simulink model "complex_iq_bw2048_out32_fixaxi_v12b"  (R2019a) (continued)
== system complex_iq_bw2048_out32_fixaxi_v12b == (continued)
      mask DialogControl t224_DualTileConfig Prompt: Dual Tile Configuration
      mask DialogControl t224_DT_adc0 Prompt: ADC 0
      mask DialogControl t224_DT_adc0_DataSettings Prompt: Data Settings
      mask DialogControl t224_DT_adc0_req_axis_clk_label Prompt: Required AXI4-Stream clock:
      mask DialogControl t224_DT_adc0_MixerSettings Prompt: MIxer Settings
      mask DialogControl t224_DT_adc0_AnalogSettings Prompt: Analog Settings
      mask DialogControl t224_DT_adc1 Prompt: ADC 1
      mask DialogControl t224_DT_adc1_DataSettings Prompt: Data Settings
      mask DialogControl t224_DT_adc1_req_axis_clk_label Prompt: Required AXI4-Stream clock:
      mask DialogControl t224_DT_adc1_MixerSettings Prompt: MIxer Settings
      mask DialogControl t224_DT_adc1_AnalogSettings Prompt: Analog Settings
      mask DialogControl Tile225_container Prompt: Tile 225
      mask DialogControl pll_clocking_config_225 Prompt: Clocking and PLL Configuration
      mask DialogControl t225_QuadTileConfig Prompt: Quad Tile Configuration
      mask DialogControl t225_ADCPair01 Prompt: ADC Pair 0,1
      mask DialogControl t225_QT_adc0 Prompt: ADC 0
      mask DialogControl t225_QT_adc0_DataSettings Prompt: Data Settings
      mask DialogControl t225_QT_adc0_req_axis_clk_label Prompt: Required AXI4-Stream clock:
      mask DialogControl t225_QT_adc0_MixerSettings Prompt: MIxer Settings
      mask DialogControl t225_QT_adc0_AnalogSettings Prompt: Analog Settings
      mask DialogControl t225_QT_adc1 Prompt: ADC 1
      mask DialogControl t225_QT_adc1_DataSettings Prompt: Data Settings
      mask DialogControl t225_QT_adc1_req_axis_clk_label Prompt: Required AXI4-Stream clock:
      mask DialogControl t225_QT_adc1_MixerSettings Prompt: MIxer Settings
      mask DialogControl t225_QT_adc1_AnalogSettings Prompt: Analog Settings
      mask DialogControl t225_ADCPair23 Prompt: ADC Pair 2,3
      mask DialogControl t225_QT_adc2 Prompt: ADC 2
      mask DialogControl t225_QT_adc2_DataSettings Prompt: Data Settings
      mask DialogControl t225_QT_adc2_req_axis_clk_label Prompt: Required AXI4-Stream clock:
      mask DialogControl t225_QT_adc2_MixerSettings Prompt: MIxer Settings
      mask DialogControl t225_QT_adc2_AnalogSettings Prompt: Analog Settings
      mask DialogControl t225_QT_adc3 Prompt: ADC 3
      mask DialogControl t225_QT_adc3_DataSettings Prompt: Data Settings
      mask DialogControl t225_QT_adc3_req_axis_clk_label Prompt: Required AXI4-Stream clock:
      mask DialogControl t225_QT_adc3_MixerSettings Prompt: MIxer Settings
      mask DialogControl t225_QT_adc3_AnalogSettings Prompt: Analog Settings
      mask DialogControl t225_DualTileConfig Prompt: Dual Tile Configuration
      mask DialogControl t225_DT_adc0 Prompt: ADC 0
      mask DialogControl t225_DT_adc0_DataSettings Prompt: Data Settings
      mask DialogControl t225_DT_adc0_req_axis_clk_label Prompt: Required AXI4-Stream clock:
      mask DialogControl t225_DT_adc0_MixerSettings Prompt: MIxer Settings
      mask DialogControl t225_DT_adc0_AnalogSettings Prompt: Analog Settings
      mask DialogControl t225_DT_adc1 Prompt: ADC 1
      mask DialogControl t225_DT_adc1_DataSettings Prompt: Data Settings
      mask DialogControl t225_DT_adc1_req_axis_clk_label Prompt: Required AXI4-Stream clock:
      mask DialogControl t225_DT_adc1_MixerSettings Prompt: MIxer Settings
      mask DialogControl t225_DT_adc1_AnalogSettings Prompt: Analog Settings
      mask DialogControl Tile226_container Prompt: Tile 226
      mask DialogControl pll_clocking_config_226 Prompt: Clocking and PLL Configuration
      mask DialogControl t226_QuadTileConfig Prompt: Quad Tile Configuration
      mask DialogControl t226_ADCPair01 Prompt: ADC Pair 0,1
      mask DialogControl t226_QT_adc0 Prompt: ADC 0
      mask DialogControl t226_QT_adc0_DataSettings Prompt: Data Settings
      mask DialogControl t226_QT_adc0_req_axis_clk_label Prompt: Required AXI4-Stream clock:
      mask DialogControl t226_QT_adc0_MixerSettings Prompt: MIxer Settings
      mask DialogControl t226_QT_adc0_AnalogSettings Prompt: Analog Settings
      mask DialogControl t226_QT_adc1 Prompt: ADC 1
      mask DialogControl t226_QT_adc1_DataSettings Prompt: Data Settings
      mask DialogControl t226_QT_adc1_req_axis_clk_label Prompt: Required AXI4-Stream clock:
      mask DialogControl t226_QT_adc1_MixerSettings Prompt: MIxer Settings
      mask DialogControl t226_QT_adc1_AnalogSettings Prompt: Analog Settings
      mask DialogControl t226_ADCPair23 Prompt: ADC Pair 2,3
      mask DialogControl t226_QT_adc2 Prompt: ADC 2
      mask DialogControl t226_QT_adc2_DataSettings Prompt: Data Settings
      mask DialogControl t226_QT_adc2_req_axis_clk_label Prompt: Required AXI4-Stream clock:
      mask DialogControl t226_QT_adc2_MixerSettings Prompt: MIxer Settings
      mask DialogControl t226_QT_adc2_AnalogSettings Prompt: Analog Settings
      mask DialogControl t226_QT_adc3 Prompt: ADC 3
      mask DialogControl t226_QT_adc3_DataSettings Prompt: Data Settings
      mask DialogControl t226_QT_adc3_req_axis_clk_label Prompt: Required AXI4-Stream clock:
      mask DialogControl t226_QT_adc3_MixerSettings Prompt: MIxer Settings
      mask DialogControl t226_QT_adc3_AnalogSettings Prompt: Analog Settings
      mask DialogControl t226_DualTileConfig Prompt: Dual Tile Configuration
      mask DialogControl t226_DT_adc0 Prompt: ADC 0
      mask DialogControl t226_DT_adc0_DataSettings Prompt: Data Settings
      mask DialogControl t226_DT_adc0_req_axis_clk_label Prompt: Required AXI4-Stream clock:
      mask DialogControl t226_DT_adc0_MixerSettings Prompt: MIxer Settings
      mask DialogControl t226_DT_adc0_AnalogSettings Prompt: Analog Settings
      mask DialogControl t226_DT_adc1 Prompt: ADC 1
      mask DialogControl t226_DT_adc1_DataSettings Prompt: Data Settings
      mask DialogControl t226_DT_adc1_req_axis_clk_label Prompt: Required AXI4-Stream clock:
      mask DialogControl t226_DT_adc1_MixerSettings Prompt: MIxer Settings
      mask DialogControl t226_DT_adc1_AnalogSettings Prompt: Analog Settings
      mask DialogControl Tile227_container Prompt: Tile 227
      mask DialogControl pll_clocking_config_227 Prompt: Clocking and PLL Configuration
      mask DialogControl t227_QuadTileConfig Prompt: Quad Tile Configuration
      mask DialogControl t227_ADCPair01 Prompt: ADC Pair 0,1
      mask DialogControl t227_QT_adc0 Prompt: ADC 0
      mask DialogControl t227_QT_adc0_DataSettings Prompt: Data Settings
      mask DialogControl t227_QT_adc0_req_axis_clk_label Prompt: Required AXI4-Stream clock:
      mask DialogControl t227_QT_adc0_MixerSettings Prompt: MIxer Settings
      mask DialogControl t227_QT_adc0_AnalogSettings Prompt: Analog Settings
      mask DialogControl t227_QT_adc1 Prompt: ADC 1
      mask DialogControl t227_QT_adc1_DataSettings Prompt: Data Settings
      mask DialogControl t227_QT_adc1_req_axis_clk_label Prompt: Required AXI4-Stream clock:
      mask DialogControl t227_QT_adc1_MixerSettings Prompt: MIxer Settings
      mask DialogControl t227_QT_adc1_AnalogSettings Prompt: Analog Settings
      mask DialogControl t227_ADCPair23 Prompt: ADC Pair 2,3
      mask DialogControl t227_QT_adc2 Prompt: ADC 2
      mask DialogControl t227_QT_adc2_DataSettings Prompt: Data Settings
      mask DialogControl t227_QT_adc2_req_axis_clk_label Prompt: Required AXI4-Stream clock:
      mask DialogControl t227_QT_adc2_MixerSettings Prompt: MIxer Settings
      mask DialogControl t227_QT_adc2_AnalogSettings Prompt: Analog Settings
      mask DialogControl t227_QT_adc3 Prompt: ADC 3
      mask DialogControl t227_QT_adc3_DataSettings Prompt: Data Settings
      mask DialogControl t227_QT_adc3_req_axis_clk_label Prompt: Required AXI4-Stream clock:
      mask DialogControl t227_QT_adc3_MixerSettings Prompt: MIxer Settings
      mask DialogControl t227_QT_adc3_AnalogSettings Prompt: Analog Settings
      mask DialogControl t227_DualTileConfig Prompt: Dual Tile Configuration
      mask DialogControl t227_DT_adc0 Prompt: ADC 0
      mask DialogControl t227_DT_adc0_DataSettings Prompt: Data Settings
      mask DialogControl t227_DT_adc0_req_axis_clk_label Prompt: Required AXI4-Stream clock:
      mask DialogControl t227_DT_adc0_MixerSettings Prompt: MIxer Settings
      mask DialogControl t227_DT_adc0_AnalogSettings Prompt: Analog Settings
      mask DialogControl t227_DT_adc1 Prompt: ADC 1
      mask DialogControl t227_DT_adc1_DataSettings Prompt: Data Settings
      mask DialogControl t227_DT_adc1_req_axis_clk_label Prompt: Required AXI4-Stream clock:
      mask DialogControl t227_DT_adc1_MixerSettings Prompt: MIxer Settings
      mask DialogControl t227_DT_adc1_AnalogSettings Prompt: Analog Settings
      mask DialogControl DACTileSelect Prompt: DAC Tiles
      mask DialogControl Tile228_container Prompt: Tile 228
      mask DialogControl pll_clocking_config_228 Prompt: Clocking and PLL Configuration
      mask DialogControl t228_QuadTileConfig Prompt: Quad Tile Configuration
      mask DialogControl t228_DACPair01 Prompt: DAC Pair 0,1
      mask DialogControl t228_QT_dac0 Prompt: DAC 0
      mask DialogControl t228_QT_dac0_DataSettings Prompt: Data Settings
      mask DialogControl t228_QT_dac0_req_axis_clk_label Prompt: Required AXI4-Stream clock:
      mask DialogControl t228_QT_dac0_MixerSettings Prompt: MIxer Settings
      mask DialogControl t228_QT_dac0_AnalogSettings Prompt: Analog Settings
      mask DialogControl t228_QT_dac1 Prompt: DAC 1
      mask DialogControl t228_QT_dac1_DataSettings Prompt: Data Settings
      mask DialogControl t228_QT_dac1_req_axis_clk_label Prompt: Required AXI4-Stream clock:
      mask DialogControl t228_QT_dac1_MixerSettings Prompt: MIxer Settings
      mask DialogControl t228_QT_dac1_AnalogSettings Prompt: Analog Settings
      mask DialogControl t228_DACPair23 Prompt: DAC Pair 2,3
      mask DialogControl t228_QT_dac2 Prompt: DAC 2
      mask DialogControl t228_QT_dac2_DataSettings Prompt: Data Settings
      mask DialogControl t228_QT_dac2_req_axis_clk_label Prompt: Required AXI4-Stream clock:
      mask DialogControl t228_QT_dac2_MixerSettings Prompt: MIxer Settings
      mask DialogControl t228_QT_dac2_AnalogSettings Prompt: Analog Settings
      mask DialogControl t228_QT_dac3 Prompt: DAC 3
      mask DialogControl t228_QT_dac3_DataSettings Prompt: Data Settings
      mask DialogControl t228_QT_dac3_req_axis_clk_label Prompt: Required AXI4-Stream clock:
      mask DialogControl t228_QT_dac3_MixerSettings Prompt: MIxer Settings
      mask DialogControl t228_QT_dac3_AnalogSettings Prompt: Analog Settings
      mask DialogControl t228_DualTileConfig Prompt: Dual Tile Configuration
      mask DialogControl t228_DT_dac0 Prompt: DAC 0
      mask DialogControl t228_DT_dac0_DataSettings Prompt: Data Settings
      mask DialogControl t228_DT_dac0_req_axis_clk_label Prompt: Required AXI4-Stream clock:
      mask DialogControl t228_DT_dac0_MixerSettings Prompt: MIxer Settings
      mask DialogControl t228_DT_dac0_AnalogSettings Prompt: Analog Settings
      mask DialogControl t228_DT_dac1 Prompt: DAC 1
      mask DialogControl t228_DT_dac1_DataSettings Prompt: Data Settings
      mask DialogControl t228_DT_dac1_req_axis_clk_label Prompt: Required AXI4-Stream clock:
      mask DialogControl t228_DT_dac1_MixerSettings Prompt: MIxer Settings
      mask DialogControl t228_DT_dac1_AnalogSettings Prompt: Analog Settings
      mask DialogControl Tile229_container Prompt: Tile 229
      mask DialogControl pll_clocking_config_229 Prompt: Clocking and PLL Configuration
      mask DialogControl t229_QuadTileConfig Prompt: Quad Tile Configuration
      mask DialogControl t229_DACPair01 Prompt: DAC Pair 0,1
      mask DialogControl t229_QT_dac0 Prompt: DAC 0
      mask DialogControl t229_QT_dac0_DataSettings Prompt: Data Settings
      mask DialogControl t229_QT_dac0_req_axis_clk_label Prompt: Required AXI4-Stream clock:
      mask DialogControl t229_QT_dac0_MixerSettings Prompt: MIxer Settings
      mask DialogControl t229_QT_dac0_AnalogSettings Prompt: Analog Settings
      mask DialogControl t229_QT_dac1 Prompt: DAC 1
      mask DialogControl t229_QT_dac1_DataSettings Prompt: Data Settings
      mask DialogControl t229_QT_dac1_req_axis_clk_label Prompt: Required AXI4-Stream clock:
      mask DialogControl t229_QT_dac1_MixerSettings Prompt: MIxer Settings
      mask DialogControl t229_QT_dac1_AnalogSettings Prompt: Analog Settings
      mask DialogControl t229_DACPair23 Prompt: DAC Pair 2,3
      mask DialogControl t229_QT_dac2 Prompt: DAC 2
      mask DialogControl t229_QT_dac2_DataSettings Prompt: Data Settings
      mask DialogControl t229_QT_dac2_req_axis_clk_label Prompt: Required AXI4-Stream clock:
      mask DialogControl t229_QT_dac2_MixerSettings Prompt: MIxer Settings
      mask DialogControl t229_QT_dac2_AnalogSettings Prompt: Analog Settings
      mask DialogControl t229_QT_dac3 Prompt: DAC 3
      mask DialogControl t229_QT_dac3_DataSettings Prompt: Data Settings
      mask DialogControl t229_QT_dac3_req_axis_clk_label Prompt: Required AXI4-Stream clock:
      mask DialogControl t229_QT_dac3_MixerSettings Prompt: MIxer Settings
      mask DialogControl t229_QT_dac3_AnalogSettings Prompt: Analog Settings
      mask DialogControl t229_DualTileConfig Prompt: Dual Tile Configuration
      mask DialogControl t229_DT_dac0 Prompt: DAC 0
      mask DialogControl t229_DT_dac0_DataSettings Prompt: Data Settings
      mask DialogControl t229_DT_dac0_req_axis_clk_label Prompt: Required AXI4-Stream clock:
      mask DialogControl t229_DT_dac0_MixerSettings Prompt: MIxer Settings
      mask DialogControl t229_DT_dac0_AnalogSettings Prompt: Analog Settings
      mask DialogControl t229_DT_dac1 Prompt: DAC 1
      mask DialogControl t229_DT_dac1_DataSettings Prompt: Data Settings
      mask DialogControl t229_DT_dac1_req_axis_clk_label Prompt: Required AXI4-Stream clock:
      mask DialogControl t229_DT_dac1_MixerSettings Prompt: MIxer Settings
      mask DialogControl t229_DT_dac1_AnalogSettings Prompt: Analog Settings
      mask DialogControl Tile230_container Prompt: Tile 230
      mask DialogControl pll_clocking_config_230 Prompt: Clocking and PLL Configuration
      mask DialogControl t230_QuadTileConfig Prompt: Quad Tile Configuration
      mask DialogControl t230_DACPair01 Prompt: DAC Pair 0,1
      mask DialogControl t230_QT_dac0 Prompt: DAC 0
      mask DialogControl t230_QT_dac0_DataSettings Prompt: Data Settings
      mask DialogControl t230_QT_dac0_req_axis_clk_label Prompt: Required AXI4-Stream clock:
      mask DialogControl t230_QT_dac0_MixerSettings Prompt: MIxer Settings
      mask DialogControl t230_QT_dac0_AnalogSettings Prompt: Analog Settings
      mask DialogControl t230_QT_dac1 Prompt: DAC 1
      mask DialogControl t230_QT_dac1_DataSettings Prompt: Data Settings
      mask DialogControl t230_QT_dac1_req_axis_clk_label Prompt: Required AXI4-Stream clock:
      mask DialogControl t230_QT_dac1_MixerSettings Prompt: MIxer Settings
      mask DialogControl t230_QT_dac1_AnalogSettings Prompt: Analog Settings
      mask DialogControl t230_DACPair23 Prompt: DAC Pair 2,3
      mask DialogControl t230_QT_dac2 Prompt: DAC 2
      mask DialogControl t230_QT_dac2_DataSettings Prompt: Data Settings
      mask DialogControl t230_QT_dac2_req_axis_clk_label Prompt: Required AXI4-Stream clock:
      mask DialogControl t230_QT_dac2_MixerSettings Prompt: MIxer Settings
      mask DialogControl t230_QT_dac2_AnalogSettings Prompt: Analog Settings
      mask DialogControl t230_QT_dac3 Prompt: DAC 3
      mask DialogControl t230_QT_dac3_DataSettings Prompt: Data Settings
      mask DialogControl t230_QT_dac3_req_axis_clk_label Prompt: Required AXI4-Stream clock:
      mask DialogControl t230_QT_dac3_MixerSettings Prompt: MIxer Settings
      mask DialogControl t230_QT_dac3_AnalogSettings Prompt: Analog Settings
      mask DialogControl t230_DualTileConfig Prompt: Dual Tile Configuration
      mask DialogControl t230_DT_dac0 Prompt: DAC 0
      mask DialogControl t230_DT_dac0_DataSettings Prompt: Data Settings
      mask DialogControl t230_DT_dac0_req_axis_clk_labe Prompt: Required AXI4-Stream clock:
      mask DialogControl t230_DT_dac0_MixerSettings Prompt: MIxer Settings
      mask DialogControl t230_DT_dac0_AnalogSettings Prompt: Analog Settings
      mask DialogControl t230_DT_dac1 Prompt: DAC 1
      mask DialogControl t230_DT_dac1_DataSettings Prompt: Data Settings
      mask DialogControl t230_DT_dac1_req_axis_clk_label Prompt: Required AXI4-Stream clock:
      mask DialogControl t230_DT_dac1_MixerSettings Prompt: MIxer Settings
      mask DialogControl t230_DT_dac1_AnalogSettings Prompt: Analog Settings
      mask DialogControl Tile231_container Prompt: Tile 231
      mask DialogControl pll_clocking_config_231 Prompt: Clocking and PLL Configuration
      mask DialogControl t231_QuadTileConfig Prompt: Quad Tile Configuration
      mask DialogControl t231_DACPair01 Prompt: DAC Pair 0,1
      mask DialogControl t231_QT_dac0 Prompt: DAC 0
      mask DialogControl t231_QT_dac0_DataSettings Prompt: Data Settings
      mask DialogControl t231_QT_dac0_req_axis_clk_label Prompt: Required AXI4-Stream clock:
      mask DialogControl t231_QT_dac0_MixerSettings Prompt: MIxer Settings
      mask DialogControl t231_QT_dac0_AnalogSettings Prompt: Analog Settings
      mask DialogControl t231_QT_dac1 Prompt: DAC 1
      mask DialogControl t231_QT_dac1_DataSettings Prompt: Data Settings
      mask DialogControl t231_QT_dac1_req_axis_clk_label Prompt: Required AXI4-Stream clock:
      mask DialogControl t231_QT_dac1_MixerSettings Prompt: MIxer Settings
      mask DialogControl t231_QT_dac1_AnalogSettings Prompt: Analog Settings
      mask DialogControl t231_DACPair23 Prompt: DAC Pair 2,3
      mask DialogControl t231_QT_dac2 Prompt: DAC 2
      mask DialogControl t231_QT_dac2_DataSettings Prompt: Data Settings
      mask DialogControl t231_QT_dac2_req_axis_clk_label Prompt: Required AXI4-Stream clock:
      mask DialogControl t231_QT_dac2_MixerSettings Prompt: MIxer Settings
      mask DialogControl t231_QT_dac2_AnalogSettings Prompt: Analog Settings
      mask DialogControl t231_QT_dac3 Prompt: DAC 3
      mask DialogControl t231_QT_dac3_DataSettings Prompt: Data Settings
      mask DialogControl t231_QT_dac3_req_axis_clk_label Prompt: Required AXI4-Stream clock:
      mask DialogControl t231_QT_dac3_MixerSettings Prompt: MIxer Settings
      mask DialogControl t231_QT_dac3_AnalogSettings Prompt: Analog Settings
      mask DialogControl t231_DualTileConfig Prompt: Dual Tile Configuration
      mask DialogControl t231_DT_dac0 Prompt: DAC 0
      mask DialogControl t231_DT_dac0_DataSettings Prompt: Data Settings
      mask DialogControl t231_DT_dac0_req_axis_clk_label Prompt: Required AXI4-Stream clock:
      mask DialogControl t231_DT_dac0_MixerSettings Prompt: MIxer Settings
      mask DialogControl t231_DT_dac0_AnalogSettings Prompt: Analog Settings
      mask DialogControl t231_DT_dac1 Prompt: DAC 1
      mask DialogControl t231_DT_dac1_DataSettings Prompt: Data Settings
      mask DialogControl t231_DT_dac1_req_axis_clk_label Prompt: Required AXI4-Stream clock:
      mask DialogControl t231_DT_dac1_MixerSettings Prompt: MIxer Settings
      mask DialogControl t231_DT_dac1_AnalogSettings Prompt: Analog Settings
      mask DialogControl SystemClocking Prompt: System Clocking
      mask DialogControl ADCClocking Prompt: ADC Tile Clocking
      mask DialogControl t224_clock_config Prompt: ADC Tile 0
      mask DialogControl t225_clock_config Prompt: ADC Tile 1
      mask DialogControl t226_clock_config Prompt: ADC Tile 2
      mask DialogControl t227_clock_config Prompt: ADC Tile 3
      mask DialogControl DACClocking Prompt: DAC Tile Clocking
      mask DialogControl t228_clock_config Prompt: DAC Tile 0
      mask DialogControl t229_clock_config Prompt: DAC Tile 1
      mask DialogControl t230_clock_config Prompt: DAC Tile 2
      mask DialogControl t231_clock_config Prompt: DAC Tile 3
  s1 : Subsystem
  s2 : Subsystem
  s3 : Subsystem
  slice_enable : xbsIndex_r4/Slice  nbits=1  boolean_output=on  mode=Lower Bit Location + Width  bit1=0  base1=MSB of Input  bit0=0  base0=LSB of Input  dbl_ovrd=off  block_type=slice
  slice_reset : xbsIndex_r4/Slice  nbits=1  boolean_output=on  mode=Lower Bit Location + Width  bit1=0  base1=MSB of Input  bit0=1  base0=LSB of Input  dbl_ovrd=off  block_type=slice
  snap_8ch : Subsystem
  snapshot_ctr0l : Subsystem  Tag=xps:sw_reg  Commented=on  AncestorBlock=xps_library/Memory/software_register  mask(swreg){io_dir=From Processor, io_delay=0, init_val=0, sample_period=1, names=[start], bitwidths=32, bin_pts=0, arith_types=2, sim_port=on, show_format=on}
      mask prompts: io_dir "I/O direction"; io_delay "I/O delay"; init_val "Initial Value"; sample_period "Sample period"; names "Bitfield names [msb...lsb]"; bitwidths "Bitfield widths"; bin_pts "Bitfield binary pts"; arith_types "Bitfield types, ufix=0, fix=1, bool=2"; sim_port "Provide sim input/output?"; show_format "Print format string?"
      mask help: A 32-bit software-accessible register. Can be divided into bitfields of varying widths and types using the fields in the block mask.
      mask init:
      | swreg_init(gcb)
      settings: AttributesFormatString=1input:b = 32 bits
      mask TypeOptions io_dir: To Processor | From Processor
      mask Callback io_dir:
      | mask_enables = get_param(gcb, 'MaskEnables');
      | mask_names = get_param(gcb, 'MaskNames');
      | if strcmp(get_param(gcb, 'io_dir'), 'To Processor'),
      |     mask_enables{ismember(mask_names, 'sample_period')} = 'off';
      | else
      |     mask_enables{ismember(mask_names, 'sample_period')} = 'on';
      | end
      | set_param(gcb, 'MaskEnables', mask_enables);
      mask DialogControl ParameterTabVar0 Prompt: Setup
  snapshot_ctrl : Subsystem  Tag=xps:sw_reg  Commented=on  AncestorBlock=xps_library/Memory/software_register  mask(swreg){io_dir=From Processor, io_delay=0, init_val=0, sample_period=1, names=[start], bitwidths=1, bin_pts=0, arith_types=2, sim_port=on, show_format=on}
      mask prompts: io_dir "I/O direction"; io_delay "I/O delay"; init_val "Initial Value"; sample_period "Sample period"; names "Bitfield names [msb...lsb]"; bitwidths "Bitfield widths"; bin_pts "Bitfield binary pts"; arith_types "Bitfield types, ufix=0, fix=1, bool=2"; sim_port "Provide sim input/output?"; show_format "Print format string?"
      mask help: A 32-bit software-accessible register. Can be divided into bitfields of varying widths and types using the fields in the block mask.
      mask init:
      | swreg_init(gcb)
      settings: AttributesFormatString=1input:b = 1 bits
      mask TypeOptions io_dir: To Processor | From Processor
      mask Callback io_dir:
      | mask_enables = get_param(gcb, 'MaskEnables');
      | mask_names = get_param(gcb, 'MaskNames');
      | if strcmp(get_param(gcb, 'io_dir'), 'To Processor'),
      |     mask_enables{ismember(mask_names, 'sample_period')} = 'off';
      | else
      |     mask_enables{ismember(mask_names, 'sample_period')} = 'on';
      | end
      | set_param(gcb, 'MaskEnables', mask_enables);
      mask DialogControl ParameterTabVar0 Prompt: Setup
  start_data : Subsystem  Tag=xps:sw_reg  AncestorBlock=xps_library/Memory/software_register  mask(swreg){io_dir=From Processor, io_delay=0, init_val=0, sample_period=1, names=reg, bitwidths=32, bin_pts=0, arith_types=0, sim_port=on, show_format=on}
      mask prompts: io_dir "I/O direction"; io_delay "I/O delay"; init_val "Initial Value"; sample_period "Sample period"; names "Bitfield names [msb...lsb]"; bitwidths "Bitfield widths"; bin_pts "Bitfield binary pts"; arith_types "Bitfield types, ufix=0, fix=1, bool=2"; sim_port "Provide sim input/output?"; show_format "Print format string?"
      mask help: A 32-bit software-accessible register. Can be divided into bitfields of varying widths and types using the fields in the block mask.
      mask init:
      | swreg_init(gcb)
      settings: AttributesFormatString=1input:uf32.0 = 32 bits
      mask TypeOptions io_dir: To Processor | From Processor
      mask Callback io_dir:
      | mask_enables = get_param(gcb, 'MaskEnables');
      | mask_names = get_param(gcb, 'MaskNames');
      | if strcmp(get_param(gcb, 'io_dir'), 'To Processor'),
      |     mask_enables{ismember(mask_names, 'sample_period')} = 'off';
      | else
      |     mask_enables{ismember(mask_names, 'sample_period')} = 'on';
      | end
      | set_param(gcb, 'MaskEnables', mask_enables);
      mask DialogControl ParameterTabVar0 Prompt: Setup
  start_data1 : Subsystem  Tag=xps:sw_reg  Commented=on  AncestorBlock=xps_library/Memory/software_register  mask(swreg){io_dir=From Processor, io_delay=0, init_val=0, sample_period=1, names=reg, bitwidths=32, bin_pts=0, arith_types=0, sim_port=on, show_format=on}
      mask prompts: io_dir "I/O direction"; io_delay "I/O delay"; init_val "Initial Value"; sample_period "Sample period"; names "Bitfield names [msb...lsb]"; bitwidths "Bitfield widths"; bin_pts "Bitfield binary pts"; arith_types "Bitfield types, ufix=0, fix=1, bool=2"; sim_port "Provide sim input/output?"; show_format "Print format string?"
      mask help: A 32-bit software-accessible register. Can be divided into bitfields of varying widths and types using the fields in the block mask.
      mask init:
      | swreg_init(gcb)
      settings: AttributesFormatString=1input:uf32.0 = 32 bits
      mask TypeOptions io_dir: To Processor | From Processor
      mask Callback io_dir:
      | mask_enables = get_param(gcb, 'MaskEnables');
      | mask_names = get_param(gcb, 'MaskNames');
      | if strcmp(get_param(gcb, 'io_dir'), 'To Processor'),
      |     mask_enables{ismember(mask_names, 'sample_period')} = 'off';
      | else
      |     mask_enables{ismember(mask_names, 'sample_period')} = 'on';
      | end
      | set_param(gcb, 'MaskEnables', mask_enables);
      mask DialogControl ParameterTabVar0 Prompt: Setup
  sum_a_b : Subsystem  Tag=xps:sw_reg  AncestorBlock=xps_library/Memory/software_register  mask(swreg){io_dir=To Processor, io_delay=0, init_val=0, sample_period=1, names=reg, bitwidths=32, bin_pts=0, arith_types=0, sim_port=on, show_format=off}
      mask prompts: io_dir "I/O direction"; io_delay "I/O delay"; init_val "Initial Value"; sample_period "Sample period"; names "Bitfield names [msb...lsb]"; bitwidths "Bitfield widths"; bin_pts "Bitfield binary pts"; arith_types "Bitfield types, ufix=0, fix=1, bool=2"; sim_port "Provide sim input/output?"; show_format "Print format string?"
      mask help: A 32-bit software-accessible register. Can be divided into bitfields of varying widths and types using the fields in the block mask.
      mask init:
      | swreg_init(gcb)
      settings: AttributesFormatString=1output
      mask TypeOptions io_dir: To Processor | From Processor
      mask Callback io_dir:
      | mask_enables = get_param(gcb, 'MaskEnables');
      | mask_names = get_param(gcb, 'MaskNames');
      | if strcmp(get_param(gcb, 'io_dir'), 'To Processor'),
      |     mask_enables{ismember(mask_names, 'sample_period')} = 'off';
      | else
      |     mask_enables{ismember(mask_names, 'sample_period')} = 'on';
      | end
      | set_param(gcb, 'MaskEnables', mask_enables);
      mask DialogControl ParameterTabVar0 Prompt: Setup
  sync_gen : Subsystem  AncestorBlock=casper_library_misc/sync_gen  mask(sync_gen){sim_acc_len=4, fft_size=2048, fft_simult_inputs=16, pfb_fir_taps=4, reorder_vec=[2 2 2], scale=1}
      mask prompts: sim_acc_len "Simulation Accumulation Length"; fft_size "FFT Size"; fft_simult_inputs "Simultaneous Inputs (FFT)"; pfb_fir_taps "Taps in pfb_fir (use 1 if no pfb_fir)"; reorder_vec "Reorder Orders (e.g. [2 2 2])"
      mask help:
      | This block computes the sync period based on system parameters as
      | described in Casper memo.  Detailed descritions of the block's
      | variables can be found in the 'Block Documentation' section of
      | the CASPER wiki.
      mask init:
      | sync_gen_init(gcb, ...
      |     'sim_acc_len', sim_acc_len, ...
      |     'fft_size', fft_size, ...
      |     'fft_simult_inputs', fft_simult_inputs,...
      |     'pfb_fir_taps', pfb_fir_taps, ...
      |     'reorder_vec', reorder_vec, ...
      |     'scale', scale);
      settings: AttributesFormatString=sim_sync_period=4096
      mask Help:
      | SyncPeriod=LCM(reorder_vec)*(acc_num)*(pfb_fir_len)*(fft_size/fft_simult_inputs)
      | 
      | See CASPER sync pulse memo for more details.
  transform2 : Subsystem
  tx_dest_ip_ram : Subsystem  Tag=xps:bram  AncestorBlock=xps_library/Memory/shared_bram  mask{arith_type=Unsigned, addr_width=2, data_width=32, reg_prim_output=on, reg_core_output=off, optimization=Minimum_Area, data_bin_pt=0, init_vals=[1 ,zeros(1,2^5-1)], sample_rate=1}
      mask prompts: arith_type "Output Data Type"; addr_width "Address width"; data_width "Data Width"; reg_prim_output "Register Primitive Output"; reg_core_output "Register Core Output"; data_bin_pt "Data Binary Point"; init_vals "Initial values (simulation only)"; sample_rate "Sample rate"
      mask init:
      | shared_bram_mask;
      mask TypeOptions arith_type: Unsigned | Signed  (2's comp)
      mask TypeOptions data_width: 8 | 16 | 32 | 64 | 128
      mask TypeOptions optimization: Minimum_Area | Low_Power
  tx_dest_port_ram : Subsystem  Tag=xps:bram  AncestorBlock=xps_library/Memory/shared_bram  mask{arith_type=Unsigned, addr_width=2, data_width=32, reg_prim_output=on, reg_core_output=off, optimization=Minimum_Area, data_bin_pt=0, init_vals=ones(1,2^5)*60000, sample_rate=1}
      mask prompts: arith_type "Output Data Type"; addr_width "Address width"; data_width "Data Width"; reg_prim_output "Register Primitive Output"; reg_core_output "Register Core Output"; data_bin_pt "Data Binary Point"; init_vals "Initial values (simulation only)"; sample_rate "Sample rate"
      mask init:
      | shared_bram_mask;
      mask TypeOptions arith_type: Unsigned | Signed  (2's comp)
      mask TypeOptions data_width: 8 | 16 | 32 | 64 | 128
      mask TypeOptions optimization: Minimum_Area | Low_Power
  vdif_on : Subsystem  Tag=xps:sw_reg  AncestorBlock=xps_library/Memory/software_register  mask(swreg){io_dir=From Processor, io_delay=0, init_val=0, sample_period=1, names=reg, bitwidths=32, bin_pts=0, arith_types=0, sim_port=on, show_format=on}
      mask prompts: io_dir "I/O direction"; io_delay "I/O delay"; init_val "Initial Value"; sample_period "Sample period"; names "Bitfield names [msb...lsb]"; bitwidths "Bitfield widths"; bin_pts "Bitfield binary pts"; arith_types "Bitfield types, ufix=0, fix=1, bool=2"; sim_port "Provide sim input/output?"; show_format "Print format string?"
      mask help: A 32-bit software-accessible register. Can be divided into bitfields of varying widths and types using the fields in the block mask.
      mask init:
      | swreg_init(gcb)
      settings: AttributesFormatString=1input:uf32.0 = 32 bits
      mask TypeOptions io_dir: To Processor | From Processor
      mask Callback io_dir:
      | mask_enables = get_param(gcb, 'MaskEnables');
      | mask_names = get_param(gcb, 'MaskNames');
      | if strcmp(get_param(gcb, 'io_dir'), 'To Processor'),
      |     mask_enables{ismember(mask_names, 'sample_period')} = 'off';
      | else
      |     mask_enables{ismember(mask_names, 'sample_period')} = 'on';
      | end
      | set_param(gcb, 'MaskEnables', mask_enables);
      mask DialogControl ParameterTabVar0 Prompt: Setup
  wires:
  pps_cnt/1(sim_out_reg) -> Terminator45/1
  Slice4/1 -> Logical1/2
  gps_pps/1 -> edge_detect5/1
  Constant25/1 -> gps_pps/1
  en_adc_sync/1(in_reg) -> Slice4/1
  Constant20/1 -> en_adc_sync/1(sim_1)
  Counter12/1 -> pps_cnt/1(out_reg)
  From85/1 -> adc2/2(adc_data)
  Constant17/1 -> snapshot_ctr0l/1(sim_1)
  snapshot_ctr0l/1(in_start) -> adc2/1(trig), adc1/1(trig)
  From83/1 -> adc1/2(adc_data)
  [adc2_i] -> snap_8ch/5(In5)
  enable7/1 -> edge_detect1/1
  [adc0_q] -> snap_8ch/2(In2)
  [adc2_q] -> snap_8ch/6(In6)
  [adc3_i] -> snap_8ch/7(In7)
  Constant31/1 -> adc_trig/1(sim_1)
  [adc3_q] -> snap_8ch/8(In8)
  [adc1_i] -> snap_8ch/3(In3)
  [adc0_i] -> snap_8ch/1(In1)
  [adc1_q] -> snap_8ch/4(In4)
  adc_trig/1(in_reg) -> enable7/1
  Logical1/1 -> Terminator9/1
  Register22/1 -> Logical2/2
  [sys_pps] -> Logical2/1
  Constant21/1 -> Register22/1
  pps_cnt1/1(sim_out_reg) -> Terminator10/1
  Counter1/1 -> pps_cnt1/1(out_reg)
  Logical2/1 -> Register34/1
  onepps/1(sync) -> [sync_gen], pipeline8/1(d)
  Register34/1 -> Register22/2
  edge_detect5/1 -> onepps/2(maser), onepps/1(gps), Counter12/1
  rfdc/1(m00_axis_tdata) -> [adc0_i]
  bitfield_snapshot1/1(arm_out) -> Terminator20/1
  bitfield_snapshot/1(arm_out) -> Terminator19/1
  snapshot_ctrl/1(in_start) -> edge_detect3/1
  Constant/1 -> bitfield_snapshot7/2(we), bitfield_snapshot6/2(we), bitfield_snapshot5/2(we), bitfield_snapshot4/2(we), bitfield_snapshot3/2(we), bitfield_snapshot2/2(we), bitfield_snapshot1/2(we), bitfield_snapshot/2(we)
  Constant4/1 -> snapshot_ctrl/1(sim_1)
  edge_detect3/1 -> bitfield_snapshot/3(trig), bitfield_snapshot4/3(trig), bitfield_snapshot5/3(trig), bitfield_snapshot6/3(trig), bitfield_snapshot7/3(trig), bitfield_snapshot3/3(trig), bitfield_snapshot2/3(trig), bitfield_snapshot1/3(trig)
  bitfield_snapshot3/1(arm_out) -> Terminator2/1
  bitfield_snapshot2/1(arm_out) -> Terminator1/1
  bitfield_snapshot4/1(arm_out) -> Terminator3/1
  bitfield_snapshot5/1(arm_out) -> Terminator4/1
  bitfield_snapshot6/1(arm_out) -> Terminator5/1
  bitfield_snapshot7/1(arm_out) -> Terminator6/1
  rfdc/5(m10_axis_tdata) -> [adc2_i]
  rfdc/6(m11_axis_tdata) -> [adc2_q]
  rfdc/7(m12_axis_tdata) -> [adc3_i]
  rfdc/8(m13_axis_tdata) -> [adc3_q]
  From3/1 -> pipeline1/1(d)
  From10/1 -> pipeline2/1(d)
  From4/1 -> pipeline6/1(d)
  From9/1 -> pipeline12/1(d)
  From8/1 -> pipeline11/1(d)
  From7/1 -> pipeline10/1(d)
  From6/1 -> pipeline9/1(d)
  From5/1 -> pipeline7/1(d)
  rfdc/4(m03_axis_tdata) -> [adc1_q]
  rfdc/3(m02_axis_tdata) -> [adc1_i]
  rfdc/2(m01_axis_tdata) -> [adc0_q]
  pipeline1/1(q) -> bitfield_snapshot/1(in_m00)
  pipeline2/1(q) -> bitfield_snapshot1/1(in_m01)
  pipeline6/1(q) -> bitfield_snapshot2/1(in_m02)
  pipeline7/1(q) -> bitfield_snapshot3/1(in_m03)
  onehundred_gbe_1/4(tx_afull) -> Terminator35/1
  Gateway Out17/1 -> Scope7/3  "endof"
  Mux3/1 -> [c_header]
  [rd_data_vld] -> Logical4/1
  Constant15/1 -> cmt_rst/1(sim_1)
  [wr_en] -> addr_11bit/1, Gateway Out41/1, Dual Port RAM14/3, Dual Port RAM17/3, Dual Port RAM16/3, Dual Port RAM15/3, Gateway Out45/1
  Dual Port RAM17/1 -> gbram16/1
  s1/1(Out1) -> Mux11/1, [sel]
  onehundred_gbe_1/2(led_rx) -> Terminator33/1
  Slice13/1 -> Gateway Out58/1, onehundred_gbe_1/4(tx_dest_ip)
  onehundred_gbe_1/11(rx_bad_frame) -> Terminator30/1
  Slice[10]1/1 -> Concat5/1
  Dual Port RAM16/2 -> Mux11/4
  onehundred_gbe_1/10(rx_end_of_frame) -> Terminator7/1
  Constant37/1 -> tx_dest_port_ram/3(we)
  Gateway Out41/1 -> Scope16/3  "wea"
  Constant69/1 -> pkt_rst_0/1(sim_1)
  [c_header] -> Gateway Out51/1, Mux12/3
  [sync_gen] -> pulse_ext/1(in)
  Gateway Out48/1 -> Scope17/4  "rd_data_vld"
  Constant1/1 -> vdif_on/1(sim_1)
  Logical4/1 -> edge_detect12/1(in), Logical/2
  Gateway Out51/1 -> Scope19/3  "c_header"
  Logical3/1 -> Delay17/1, edge_detect9/1(in)
  Logical/1 -> edge_detect11/1(in)
  Register7/1 -> Dual Port RAM16/1, Dual Port RAM17/1, Dual Port RAM15/1, Gateway Out39/1, Dual Port RAM14/1  "rd_en"
  concatenate/1 -> Mux3/3
  [dout] -> Delay38/1
  addr_11bit/1 -> Gateway Out46/1, Register7/1, Slice[10]/1
  cmt_rst/1(in_reg) -> pipeline20/1(d)
  Delay17/1 -> Logical7/1
  Concat5/1 -> Dual Port RAM17/4, Dual Port RAM16/4, Dual Port RAM15/4, Gateway Out42/1, Dual Port RAM14/4
  [generate_header] -> addr6/2, addr4/2, edge_detect4/1
  onehundred_gbe_1/5(tx_overflow) -> Terminator36/1
  Slice[10]/1 -> Register10/1, Register10/2
  [rd_data_vld] -> Gateway Out48/1
  Constant36/1 -> tx_dest_ip_ram/3(we)
  onehundred_gbe_1/6(rx_data) -> Terminator26/1
  Logical7/1 -> Gateway Out56/1, Gateway Out60/1, onehundred_gbe_1/3(tx_valid)
  Constant62/1 -> Dual Port RAM17/5  "dinb"
  pipeline20/1(q) -> enable6/1
  Dual Port RAM16/1 -> gbram24/1
  Gateway Out47/1 -> Scope18/3
  edge_detect11/1(out) -> [generate_header]
  Gateway Out40/1 -> Scope16/2  "dina"
  edge_detect9/1(out) -> Gateway Out54/1, Gateway Out57/1, onehundred_gbe_1/6(tx_end_of_frame)
  Constant56/1 -> Dual Port RAM14/5  "dinb"
  Constant34/1 -> tx_dest_port_ram/2(data_in)
  pkt_rst_0/1(in_reg) -> pipeline47/1(d)
  [sel] -> Gateway Out43/1
  Delay41/1 -> Slice12/1
  Constant61/1 -> Dual Port RAM16/6  "web"
  onehundred_gbe_1/13(rx_dest_ip) -> Terminator27/1
  Constant35/1 -> tx_dest_ip_ram/2(data_in)
  Gateway Out59/1 -> Scope22/2  "tx_dest_port"
  onehundred_gbe_1/7(rx_valid) -> Terminator31/1
  Dual Port RAM17/2 -> Mux11/5
  Slice[7]/1 -> Delay36/1
  Gateway Out16/1 -> Scope7/2  "frame_p"
  Gateway Out45/1 -> Scope18/1
  Gateway Out56/1 -> Scope21/2  "tx_valid"
  onehundred_gbe_1/14(rx_dest_port) -> Terminator29/1
  [tx_dest_ip] -> Relational/1, Delay40/1
  edge_detect12/1(out) -> [print_header]
  [print_header] -> Logical3/2, Gateway Out53/1
  Gateway Out44/1 -> Scope17/2  "dout"
  Constant58/1 -> Dual Port RAM14/6  "web"
  Constant67/1 -> onehundred_gbe_1/9(debug_rst)
  Constant76/1 -> Relational3/2
  Gateway Out57/1 -> Scope21/3  "tx_end_of_frame"
  Dual Port RAM15/1 -> gbram22/1
  Gateway Out60/1 -> Scope22/3  "tx_valid"
  Concat4/1 -> Dual Port RAM16/2, Dual Port RAM17/2  "dina"
  Gateway Out55/1 -> Scope21/1  "tx_data"
  Delay38/1 -> Gateway Out50/1, Mux12/2
  [sel] -> Delay9/1
  Gateway Out46/1 -> Scope18/2
  Gateway Out49/1 -> Scope19/1  "print_header"
  [print_header] -> Gateway Out49/1, Mux12/1
  edge_detect4/1 -> hdr0/2(start_frame), Gateway Out16/1
  pipeline30/1(q) -> Slice2/1
  pipeline47/1(q) -> Slice14/1
  Gateway Out39/1 -> Scope16/1  "addr1"
  Gateway Out52/1 -> Scope20/1  "rd_data_vld"
  Dual Port RAM15/2 -> Mux11/3
  Constant64/1 -> onehundred_gbe_1/10(tx_byte_enable)
  Slice2/1 -> Mux3/1
  Gateway Out43/1 -> Scope17/3  "sel"
  enable6/1 -> addr6/1, addr4/1
  Constant65/1 -> onehundred_gbe_1/7(rx_ack)
  Slice[6:0]/1 -> Concat5/2
  [sel] -> Delay12/1
  Delay12/1 -> tx_dest_ip_ram/1(addr)
  Delay39/1 -> Gateway Out52/1, Logical3/1
  Delay9/1 -> tx_dest_port_ram/1(addr)
  [dout] -> Gateway Out44/1
  onehundred_gbe_1/1(led_up) -> Terminator32/1
  Relational3/1 -> Logical/1
  Constant77/1 -> Logical4/2
  Constant63/1 -> Dual Port RAM17/6  "web"
  [tx_dest_port] -> Delay41/1
  Gateway Out42/1 -> Scope17/1  "addrb"
  Relational/1 -> Logical7/2
  hdr0/1(hdr) -> concatenate/2, Gateway Out17/1
  onehundred_gbe_1/12(rx_overrun) -> Terminator25/1
  Constant30/1 -> Relational/2  "web"
  Gateway Out58/1 -> Scope22/1  "tx_dest_ip"
  edge_detect8/1(out) -> hdr0/1(sync_in), Gateway Out15/1
  pulse_ext/1(out) -> edge_detect8/1(in)
  addr4/1 -> concatenate/1
  onehundred_gbe_1/8(rx_source_ip) -> Terminator28/1
  onehundred_gbe_1/3(led_tx) -> Terminator34/1
  Delay36/1 -> [rd_data_vld]
  addr6/1 -> Mux3/2
  vdif_on/1(in_reg) -> pipeline30/1(d)
  Constant60/1 -> Dual Port RAM16/5  "dinb"
  [sel] -> Relational3/1
  Gateway Out15/1 -> Scope7/1  "sync"
  Delay35/1 -> Gateway Out55/1, onehundred_gbe_1/2(tx_data)
  onehundred_gbe_1/9(rx_source_port) -> Terminator37/1
  Gateway Out53/1 -> Scope20/2  "print_header"
  Dual Port RAM14/2 -> Mux11/2
  Mux12/1 -> Delay35/1
  Mux11/1 -> [dout]
  Constant57/1 -> Dual Port RAM15/6
  Delay40/1 -> Slice13/1
  Gateway Out50/1 -> Scope19/2  "dout"
  Constant68/1 -> onehundred_gbe_1/8(rx_overrun_ack)
  tx_dest_port_ram/1(data_out) -> [tx_dest_port]
  Slice12/1 -> Gateway Out59/1, onehundred_gbe_1/5(tx_dest_port)
  Constant55/1 -> Dual Port RAM15/5
  addr_11bit1/1 -> Gateway Out47/1, Slice[7]/1, s1/1(In1), Slice[6:0]/1, Slice[10]1/1
  Gateway Out54/1 -> Scope20/3  "tx_end_of_frame"
  [rd_data_vld] -> Delay39/1
  Slice14/1 -> edge_detect10/1(in)
  tx_dest_ip_ram/1(data_out) -> [tx_dest_ip]
  edge_detect10/1(out) -> onehundred_gbe_1/1(rst)
  [data_0] -> Gateway Out40/1, Dual Port RAM14/2  "dina"
  Register10/1 -> addr_11bit1/1  "rd_en"
  Dual Port RAM14/1 -> gbram23/1
  pipeline8/1(q) -> pipeline18/1(d), [sys_pps]
  pipeline9/1(q) -> bitfield_snapshot4/1(in_m20)
  pipeline10/1(q) -> bitfield_snapshot5/1(in_m21)
  pipeline11/1(q) -> bitfield_snapshot6/1(in_m22)
  pipeline12/1(q) -> bitfield_snapshot7/1(in_m23)
  pipeline18/1(q) -> led1/1(In1)
  pipeline19/1(q) -> bus_expand_14bit/1(bus_in)
  pipeline21/1(q) -> bus_expand_14bit1/1(bus_in)
  [adc0_q] -> pipeline21/1(d)
  [adc0_i] -> pipeline19/1(d)
  bus_expand_14bit/1(msb_out16) -> dec_fir1/2(real1)
  bus_expand_14bit/2(out15) -> dec_fir1/4(real2)
  bus_expand_14bit/3(out14) -> dec_fir1/6(real3)
  bus_expand_14bit/4(out13) -> dec_fir1/8(real4)
  bus_expand_14bit/5(out12) -> dec_fir1/10(real5)
  bus_expand_14bit/6(out11) -> dec_fir1/12(real6)
  bus_expand_14bit/7(out10) -> dec_fir1/14(real7)
  bus_expand_14bit/8(out9) -> dec_fir1/16(real8)
  bus_expand_14bit1/1(msb_out16) -> dec_fir1/3(imag1)
  bus_expand_14bit1/2(out15) -> dec_fir1/5(imag2)
  bus_expand_14bit1/3(out14) -> dec_fir1/7(imag3)
  bus_expand_14bit1/4(out13) -> dec_fir1/9(imag4)
  bus_expand_14bit1/5(out12) -> dec_fir1/11(imag5)
  bus_expand_14bit1/6(out11) -> dec_fir1/13(imag6)
  bus_expand_14bit1/7(out10) -> dec_fir1/15(imag7)
  bus_expand_14bit1/8(out9) -> dec_fir1/17(imag8)
  [sync_gen] -> dec_fir1/1(sync_in)
  b1_bram/1(data_out) -> gbram103/1
  s2/1(Out1) -> b1_bram/1(addr)
  s2/2(Out2) -> b1_bram/3(we)
  b2_bram/1(data_out) -> gbram3/1
  s3/1(Out1) -> b2_bram/1(addr)
  edge_detect1/1 -> snap_8ch/9(trig), s3/1(In1), s2/1(In1), Register22/3
  s3/2(Out2) -> b2_bram/3(we)
  bus_expand1/7(out10) -> dec_fir2/8(real4)
  bus_expand1/3(out14) -> dec_fir2/4(real2)
  pipeline23/1(q) -> bus_create1/2(in2)
  bus_expand1/10(out7) -> dec_fir2/11(imag5)
  bus_expand1/2(out15) -> dec_fir2/3(imag1)
  bus_expand1/14(out3) -> dec_fir2/15(imag7)
  pipeline22/1(q) -> bus_create1/1(in1)
  bus_expand1/12(out5) -> dec_fir2/13(imag6)
  bus_expand1/16(lsb_out1) -> dec_fir2/17(imag8)
  bus_expand1/1(msb_out16) -> dec_fir2/2(real1)
  bus_expand1/5(out12) -> dec_fir2/6(real3)
  bus_expand1/13(out4) -> dec_fir2/14(real7)
  bus_expand1/4(out13) -> dec_fir2/5(imag2)
  bus_expand1/15(out2) -> dec_fir2/16(real8)
  From28/1 -> pipeline22/1(d)
  From30/1 -> dec_fir2/1(sync_in)
  From29/1 -> pipeline23/1(d)
  bus_expand1/11(out6) -> dec_fir2/12(real6)
  bus_expand1/9(out8) -> dec_fir2/10(real5)
  munge2/1(dout) -> bus_expand1/1(bus_in)
  bus_create1/1(bus_out) -> munge2/1(din)
  dec_fir2/1(sync_out) -> gbram2/1
  bus_expand1/8(out9) -> dec_fir2/9(imag4)
  bus_expand1/6(out11) -> dec_fir2/7(imag3)
  bus_expand_14bit3/2(out15) -> dec_fir3/5(imag2)
  bus_expand_14bit3/8(out9) -> dec_fir3/17(imag8)
  bus_expand_14bit3/3(out14) -> dec_fir3/7(imag3)
  bus_expand_14bit2/3(out14) -> dec_fir3/6(real3)
  bus_expand_14bit3/6(out11) -> dec_fir3/13(imag6)
  bus_expand_14bit3/5(out12) -> dec_fir3/11(imag5)
  bus_expand_14bit2/4(out13) -> dec_fir3/8(real4)
  bus_expand_14bit2/2(out15) -> dec_fir3/4(real2)
  bus_expand_14bit3/1(msb_out16) -> dec_fir3/3(imag1)
  bus_expand_14bit3/4(out13) -> dec_fir3/9(imag4)
  bus_expand_14bit2/8(out9) -> dec_fir3/16(real8)
  [sync_gen] -> dec_fir3/1(sync_in)
  bus_expand_14bit3/7(out10) -> dec_fir3/15(imag7)
  dec_fir3/2(dout) -> Concat2/2, pipeline27/1(d)
  bus_expand_14bit2/1(msb_out16) -> dec_fir3/2(real1)
  bus_expand_14bit2/7(out10) -> dec_fir3/14(real7)
  bus_expand_14bit2/5(out12) -> dec_fir3/10(real5)
  dec_fir3/1(sync_out) -> gbram4/1
  bus_expand_14bit2/6(out11) -> dec_fir3/12(real6)
  pipeline25/1(q) -> bus_expand_14bit3/1(bus_in)
  [adc1_i] -> pipeline24/1(d)
  [adc1_q] -> pipeline25/1(d)
  pipeline24/1(q) -> bus_expand_14bit2/1(bus_in)
  Concat2/1 -> Register18/1, [data_0]
  dec_fir1/2(dout) -> Concat2/1, pipeline26/1(d)
  pipeline26/1(q) -> b1_bram/2(data_in)
  pipeline27/1(q) -> b2_bram/2(data_in)
  Constant3/1 -> Concat6/4, Concat6/3, Concat6/2, Concat6/1  "web"
  Concat6/1 -> Concat4/1, Concat4/2
  Logical8/1 -> Register1/1
  Slice1/1 -> Register1/2
  start_data1/1(in_reg) -> Slice1/1
  Register1/1 -> Logical9/1, Logical9/2
  Constant2/1 -> start_data1/1(sim_1)
  From2/1 -> Logical8/2, Logical8/1
  Logical9/1 -> Goto10/1
  Logical6/1 -> [wr_en]
  Register9/1 -> Logical6/2
  [sync_gen] -> Logical5/1
  Logical5/1 -> Register9/2, Logical6/1, Register9/1
  Constant5/1 -> start_data/1(sim_1)
  start_data/1(in_reg) -> enable1/1
  enable1/1 -> Logical5/2
  Constant6/1 -> counter_ctrl/1(sim_1)
  b_sim/1 -> bin/1(sim_1)
  counter_value/1(sim_out_reg) -> Scope/1
  AddSub/1 -> sum_a_b/1(out_reg)
  ain/1(in_reg) -> AddSub/1
  counter_led1/1 -> counter_value/1(out_reg)
  a_sim/1 -> ain/1(sim_1)
  counter_ctrl/1(in_reg) -> slice_reset/1, slice_enable/1
  slice_reset/1 -> counter_led1/1
  bin/1(in_reg) -> AddSub/2
  slice_enable/1 -> counter_led1/2
  sum_a_b/1(sim_out_reg) -> Scope1/1
  sync_gen/1(sync_out) -> Terminator8/1
  Register18/1 -> transform2/1(In1)
  Register31/1 -> Register2/1
  transform2/1(Out1) -> [data_offset]
  Register2/1 -> Register35/1
  dec_fir1/1(sync_out) -> Register31/1
  Register35/1 -> [sys_out]
  [data_offset] -> Dual Port RAM15/2  "dina"
